simulink model "HvacPartRecircCtrl"  (R2022b)
settings: Solver=FixedStepDiscrete  StartTime=0.0  StopTime=10.0  FixedStep=0.2  RelTol=1e-3
settings Model: Creator=blue.ge  LastModifiedBy=blue.ge
settings DataIOCC: SaveFormat=StructureWithTime
settings OptimizationCC: DefaultParameterBehavior=Inlined  EnhancedBackFolding=on  PassReuseOutputArgsAs=Individual arguments  ZeroExternalMemoryAtStartup=off  ZeroInternalMemoryAtStartup=off  OptimizeBlockOrder=speed  DifferentSizesBufferReuse=on  OptimizationCustomize=off  EfficientTunableParamExpr=on
settings DebuggingCC: ParameterTunabilityLossMsg=error
settings ModelReferenceCC: ModelReferenceNumInstancesAllowed=Single
settings SFSimCC: MATLABDynamicMemAlloc=off
settings RTWCC: Description=AUTOSAR  SystemTargetFile=autosar.tlc  GenCodeOnly=on  TemplateMakefile=ert_default_tmf
settings CodeAppCC: ForceParamTrailComments=on  IgnoreCustomStorageClasses=off  OperatorAnnotations=on  SimulinkDataObjDesc=on  SFDataObjDesc=on  InsertBlockDesc=on
settings STFCustomTargetCC: TargetLangStandard=C99 (ISO)  MultiwordLength=256  ParMdlRefBuildCompliant=on  CompOptLevelCompliant=on  IncludeMdlTerminateFcn=off  CombineOutputUpdateFcns=on  SuppressErrorStatus=on  ERTCustomFileBanners=on  SupportNonFinite=off  SupportComplex=off  SupportContinuousTime=off  SupportNonInlinedSFcns=off  RemoveResetFunc=on  MATLABClassNameForMDSCustomization=Simulink.SoftwareTarget.ERTCustomization  AutosarCompliant=on  UseToolchainInfoCompliant=on  LookupTableObjectStructAxisOrder=2,1,3,4,...  LUTObjectStructOrderEvenSpacing=Size,Table,Breakpoints  SystemTargetFile=autosar.tlc
settings ERTTargetCC: TargetLangStandard=C99 (ISO)  ConcurrentExecutionCompliant=off  IncludeMdlTerminateFcn=off  SuppressErrorStatus=on  SupportNonFinite=off  SupportComplex=off  ModelStepFunctionPrototypeControlCompliant=off  CPPClassGenCompliant=off  AutosarCompliant=on  LookupTableObjectStructAxisOrder=2,1,3,4,...  LUTObjectStructOrderEvenSpacing=Size,Table,Breakpoints  InlinedParameterPlacement=NonHierarchical  ERTCustomFileTemplate=example_file_process.tlc

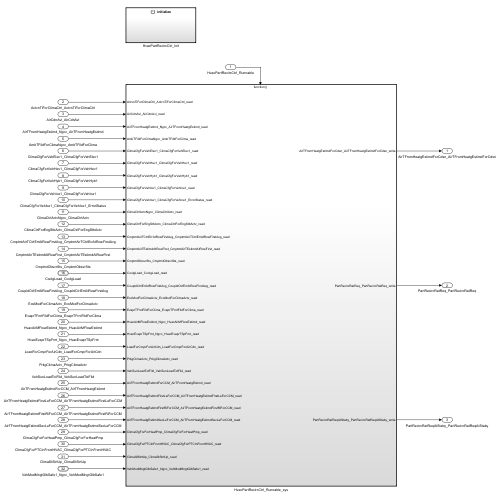
[thumbnail: Simulink's stored preview of the top-level diagram]
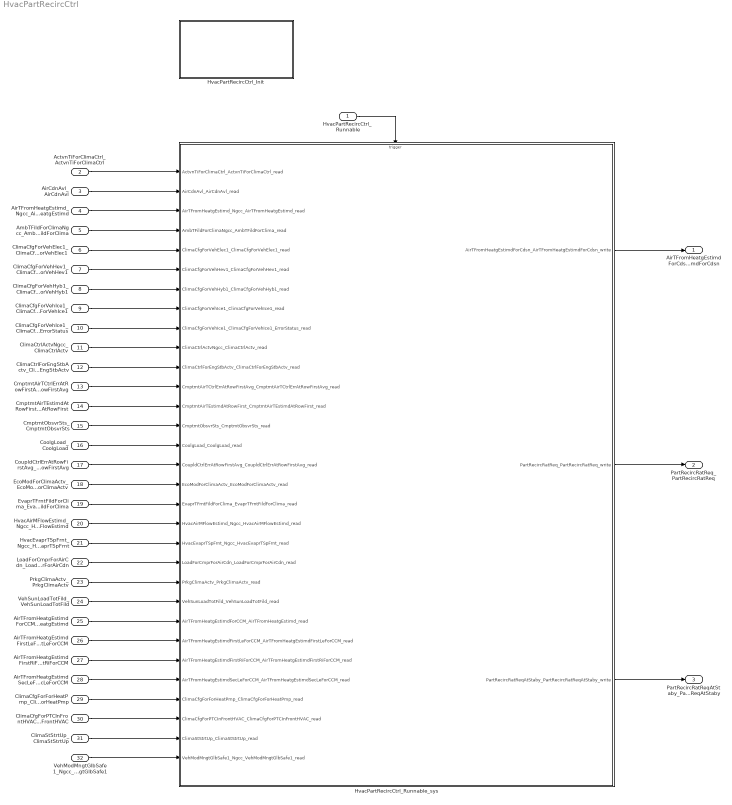
== system HvacPartRecircCtrl ==
  HvacPartRecircCtrl_Runnable : Inport  SampleTime=0.2  OutputFunctionCall=on
  ActvnTiForClimaCtrl_ActvnTiForClimaCtrl : Inport  Port=2  OutDataTypeStr=Ti33  PortDimensions=1
  AirCdnAvl_AirCdnAvl : Inport  Port=3  OutDataTypeStr=Boolean  PortDimensions=1
  AirTFromHeatgEstimd_Ngcc_AirTFromHeatgEstimd : Inport  Port=4  OutDataTypeStr=T30  PortDimensions=1
  AmbTFildForClimaNgcc_AmbTFildForClima : Inport  Port=5  OutDataTypeStr=T30  PortDimensions=1
  ClimaCfgForVehElec1_ClimaCfgForVehElec1 : Inport  Port=6  OutDataTypeStr=Boolean  PortDimensions=1
  ClimaCfgForVehHev1_ClimaCfgForVehHev1 : Inport  Port=7  OutDataTypeStr=Boolean  PortDimensions=1
  ClimaCfgForVehHyb1_ClimaCfgForVehHyb1 : Inport  Port=8  OutDataTypeStr=Boolean  PortDimensions=1
  ClimaCfgForVehIce1_ClimaCfgForVehIce1 : Inport  Port=9  OutDataTypeStr=Boolean  PortDimensions=1
  ClimaCfgForVehIce1_ClimaCfgForVehIce1_ErrorStatus : Inport  Port=10  OutDataTypeStr=uint8  PortDimensions=1
  ClimaCtrlActvNgcc_ClimaCtrlActv : Inport  Port=11  OutDataTypeStr=Boolean  PortDimensions=1
  ClimaCtrlForEngStbActv_ClimaCtrlForEngStbActv : Inport  Port=12  OutDataTypeStr=Boolean  PortDimensions=1
  CmptmtAirTCtrlErrAtRowFirstAvg_CmptmtAirTCtrlErrAtRowFirstAvg : Inport  Port=13  OutDataTypeStr=T30  PortDimensions=1
  CmptmtAirTEstimdAtRowFirst_CmptmtAirTEstimdAtRowFirst : Inport  Port=14  OutDataTypeStr=T30  PortDimensions=1
  CmptmtObsvrSts_CmptmtObsvrSts : Inport  Port=15  OutDataTypeStr=Enum: ObsvrStsForCmptmt  PortDimensions=1
  CoolgLoad_CoolgLoad : Inport  Port=16  OutDataTypeStr=Bus: CoolgLoad1  PortDimensions=1  BusOutputAsStruct=on
  CoupldCtrlErrAtRowFirstAvg_CoupldCtrlErrAtRowFirstAvg : Inport  Port=17  OutDataTypeStr=T30  PortDimensions=1
  EcoModForClimaActv_EcoModForClimaActv : Inport  Port=18  OutDataTypeStr=Enum: OnOff1  PortDimensions=1
  EvaprTFrntFildForClima_EvaprTFrntFildForClima : Inport  Port=19  OutDataTypeStr=T30  PortDimensions=1
  HvacAirMFlowEstimd_Ngcc_HvacAirMFlowEstimd : Inport  Port=20  OutMin=0  OutMax=1000  OutDataTypeStr=MFlow1  PortDimensions=1
  HvacEvaprTSpFrnt_Ngcc_HvacEvaprTSpFrnt : Inport  Port=21  OutDataTypeStr=HvacEvaprTSp  PortDimensions=1
  LoadForCmprForAirCdn_LoadForCmprForAirCdn : Inport  Port=22  OutDataTypeStr=Perc4  PortDimensions=1
  PrkgClimaActv_PrkgClimaActv : Inport  Port=23  OutDataTypeStr=Enum: PrkgClimaActv  PortDimensions=1
  VehSunLoadTotFild_VehSunLoadTotFild : Inport  Port=24  OutDataTypeStr=SunLoad30  PortDimensions=1
  AirTFromHeatgEstimdForCCM_AirTFromHeatgEstimd : Inport  Port=25  OutDataTypeStr=T30  PortDimensions=1
  AirTFromHeatgEstimdFirstLeForCCM_AirTFromHeatgEstimdFirstLeForCCM : Inport  Port=26  OutDataTypeStr=T30  PortDimensions=1
  AirTFromHeatgEstimdFirstRiForCCM_AirTFromHeatgEstimdFirstRiForCCM : Inport  Port=27  OutDataTypeStr=T30  PortDimensions=1
  AirTFromHeatgEstimdSecLeForCCM_AirTFromHeatgEstimdSecLeForCCM : Inport  Port=28  OutDataTypeStr=T30  PortDimensions=1
  ClimaCfgForForHeatPmp_ClimaCfgForForHeatPmp : Inport  Port=29  OutDataTypeStr=Enum: CarCfgForHeatPmpTyp  PortDimensions=1
  ClimaCfgForPTCInFrontHVAC_ClimaCfgForPTCInFrontHVAC : Inport  Port=30  OutDataTypeStr=Enum: FrontHeaterExchanger  PortDimensions=1
  ClimaStStrtUp_ClimaStStrtUp : Inport  Port=31  OutDataTypeStr=Enum: ClimaStStrtUp  PortDimensions=1
  VehModMngtGlbSafe1_Ngcc_VehModMngtGlbSafe1 : Inport  Port=32  OutDataTypeStr=Bus: VehModMngtGlbSafe1  PortDimensions=1  BusOutputAsStruct=on
  HvacPartRecircCtrl_Init : Subsystem
  HvacPartRecircCtrl_Runnable_sys : Subsystem
  AirTFromHeatgEstimdForCdsn_AirTFromHeatgEstimdForCdsn : Outport  OutDataTypeStr=T30  PortDimensions=1
  PartRecircRatReq_PartRecircRatReq : Outport  Port=2  OutDataTypeStr=Perc30  PortDimensions=1
  PartRecircRatReqAtStaby_PartRecircRatReqAtStaby : Outport  Port=3  OutDataTypeStr=Perc30  PortDimensions=1
  wires:
  ActvnTiForClimaCtrl_ActvnTiForClimaCtrl/1 -> HvacPartRecircCtrl_Runnable_sys/1(ActvnTiForClimaCtrl_ActvnTiForClimaCtrl_read)
  AirCdnAvl_AirCdnAvl/1 -> HvacPartRecircCtrl_Runnable_sys/2(AirCdnAvl_AirCdnAvl_read)
  AirTFromHeatgEstimd_Ngcc_AirTFromHeatgEstimd/1 -> HvacPartRecircCtrl_Runnable_sys/3(AirTFromHeatgEstimd_Ngcc_AirTFromHeatgEstimd_read)
  AmbTFildForClimaNgcc_AmbTFildForClima/1 -> HvacPartRecircCtrl_Runnable_sys/4(AmbTFildForClimaNgcc_AmbTFildForClima_read)
  ClimaCfgForVehElec1_ClimaCfgForVehElec1/1 -> HvacPartRecircCtrl_Runnable_sys/5(ClimaCfgForVehElec1_ClimaCfgForVehElec1_read)
  ClimaCfgForVehHev1_ClimaCfgForVehHev1/1 -> HvacPartRecircCtrl_Runnable_sys/6(ClimaCfgForVehHev1_ClimaCfgForVehHev1_read)
  ClimaCfgForVehHyb1_ClimaCfgForVehHyb1/1 -> HvacPartRecircCtrl_Runnable_sys/7(ClimaCfgForVehHyb1_ClimaCfgForVehHyb1_read)
  ClimaCfgForVehIce1_ClimaCfgForVehIce1/1 -> HvacPartRecircCtrl_Runnable_sys/8(ClimaCfgForVehIce1_ClimaCfgForVehIce1_read)
  ClimaCfgForVehIce1_ClimaCfgForVehIce1_ErrorStatus/1 -> HvacPartRecircCtrl_Runnable_sys/9(ClimaCfgForVehIce1_ClimaCfgForVehIce1_ErrorStatus_read)
  ClimaCtrlActvNgcc_ClimaCtrlActv/1 -> HvacPartRecircCtrl_Runnable_sys/10(ClimaCtrlActvNgcc_ClimaCtrlActv_read)
  ClimaCtrlForEngStbActv_ClimaCtrlForEngStbActv/1 -> HvacPartRecircCtrl_Runnable_sys/11(ClimaCtrlForEngStbActv_ClimaCtrlForEngStbActv_read)
  CmptmtAirTCtrlErrAtRowFirstAvg_CmptmtAirTCtrlErrAtRowFirstAvg/1 -> HvacPartRecircCtrl_Runnable_sys/12(CmptmtAirTCtrlErrAtRowFirstAvg_CmptmtAirTCtrlErrAtRowFirstAvg_read)
  CmptmtAirTEstimdAtRowFirst_CmptmtAirTEstimdAtRowFirst/1 -> HvacPartRecircCtrl_Runnable_sys/13(CmptmtAirTEstimdAtRowFirst_CmptmtAirTEstimdAtRowFirst_read)
  CmptmtObsvrSts_CmptmtObsvrSts/1 -> HvacPartRecircCtrl_Runnable_sys/14(CmptmtObsvrSts_CmptmtObsvrSts_read)
  CoolgLoad_CoolgLoad/1 -> HvacPartRecircCtrl_Runnable_sys/15(CoolgLoad_CoolgLoad_read)
  CoupldCtrlErrAtRowFirstAvg_CoupldCtrlErrAtRowFirstAvg/1 -> HvacPartRecircCtrl_Runnable_sys/16(CoupldCtrlErrAtRowFirstAvg_CoupldCtrlErrAtRowFirstAvg_read)
  EcoModForClimaActv_EcoModForClimaActv/1 -> HvacPartRecircCtrl_Runnable_sys/17(EcoModForClimaActv_EcoModForClimaActv_read)
  EvaprTFrntFildForClima_EvaprTFrntFildForClima/1 -> HvacPartRecircCtrl_Runnable_sys/18(EvaprTFrntFildForClima_EvaprTFrntFildForClima_read)
  HvacAirMFlowEstimd_Ngcc_HvacAirMFlowEstimd/1 -> HvacPartRecircCtrl_Runnable_sys/19(HvacAirMFlowEstimd_Ngcc_HvacAirMFlowEstimd_read)
  HvacEvaprTSpFrnt_Ngcc_HvacEvaprTSpFrnt/1 -> HvacPartRecircCtrl_Runnable_sys/20(HvacEvaprTSpFrnt_Ngcc_HvacEvaprTSpFrnt_read)
  LoadForCmprForAirCdn_LoadForCmprForAirCdn/1 -> HvacPartRecircCtrl_Runnable_sys/21(LoadForCmprForAirCdn_LoadForCmprForAirCdn_read)
  PrkgClimaActv_PrkgClimaActv/1 -> HvacPartRecircCtrl_Runnable_sys/22(PrkgClimaActv_PrkgClimaActv_read)
  VehSunLoadTotFild_VehSunLoadTotFild/1 -> HvacPartRecircCtrl_Runnable_sys/23(VehSunLoadTotFild_VehSunLoadTotFild_read)
  AirTFromHeatgEstimdForCCM_AirTFromHeatgEstimd/1 -> HvacPartRecircCtrl_Runnable_sys/24(AirTFromHeatgEstimdForCCM_AirTFromHeatgEstimd_read)
  AirTFromHeatgEstimdFirstLeForCCM_AirTFromHeatgEstimdFirstLeForCCM/1 -> HvacPartRecircCtrl_Runnable_sys/25(AirTFromHeatgEstimdFirstLeForCCM_AirTFromHeatgEstimdFirstLeForCCM_read)
  AirTFromHeatgEstimdFirstRiForCCM_AirTFromHeatgEstimdFirstRiForCCM/1 -> HvacPartRecircCtrl_Runnable_sys/26(AirTFromHeatgEstimdFirstRiForCCM_AirTFromHeatgEstimdFirstRiForCCM_read)
  AirTFromHeatgEstimdSecLeForCCM_AirTFromHeatgEstimdSecLeForCCM/1 -> HvacPartRecircCtrl_Runnable_sys/27(AirTFromHeatgEstimdSecLeForCCM_AirTFromHeatgEstimdSecLeForCCM_read)
  ClimaCfgForForHeatPmp_ClimaCfgForForHeatPmp/1 -> HvacPartRecircCtrl_Runnable_sys/28(ClimaCfgForForHeatPmp_ClimaCfgForForHeatPmp_read)
  ClimaCfgForPTCInFrontHVAC_ClimaCfgForPTCInFrontHVAC/1 -> HvacPartRecircCtrl_Runnable_sys/29(ClimaCfgForPTCInFrontHVAC_ClimaCfgForPTCInFrontHVAC_read)
  ClimaStStrtUp_ClimaStStrtUp/1 -> HvacPartRecircCtrl_Runnable_sys/30(ClimaStStrtUp_ClimaStStrtUp_read)
  VehModMngtGlbSafe1_Ngcc_VehModMngtGlbSafe1/1 -> HvacPartRecircCtrl_Runnable_sys/31(VehModMngtGlbSafe1_Ngcc_VehModMngtGlbSafe1_read)
  HvacPartRecircCtrl_Runnable_sys/1(AirTFromHeatgEstimdForCdsn_AirTFromHeatgEstimdForCdsn_write) -> AirTFromHeatgEstimdForCdsn_AirTFromHeatgEstimdForCdsn/1
  HvacPartRecircCtrl_Runnable_sys/2(PartRecircRatReq_PartRecircRatReq_write) -> PartRecircRatReq_PartRecircRatReq/1
  HvacPartRecircCtrl_Runnable_sys/3(PartRecircRatReqAtStaby_PartRecircRatReqAtStaby_write) -> PartRecircRatReqAtStaby_PartRecircRatReqAtStaby/1
  HvacPartRecircCtrl_Runnable/1 -> HvacPartRecircCtrl_Runnable_sys/Trigger

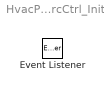
== system HvacPartRecircCtrl/HvacPartRecircCtrl_Init ==
  Event Listener : EventListener  EventName=reset

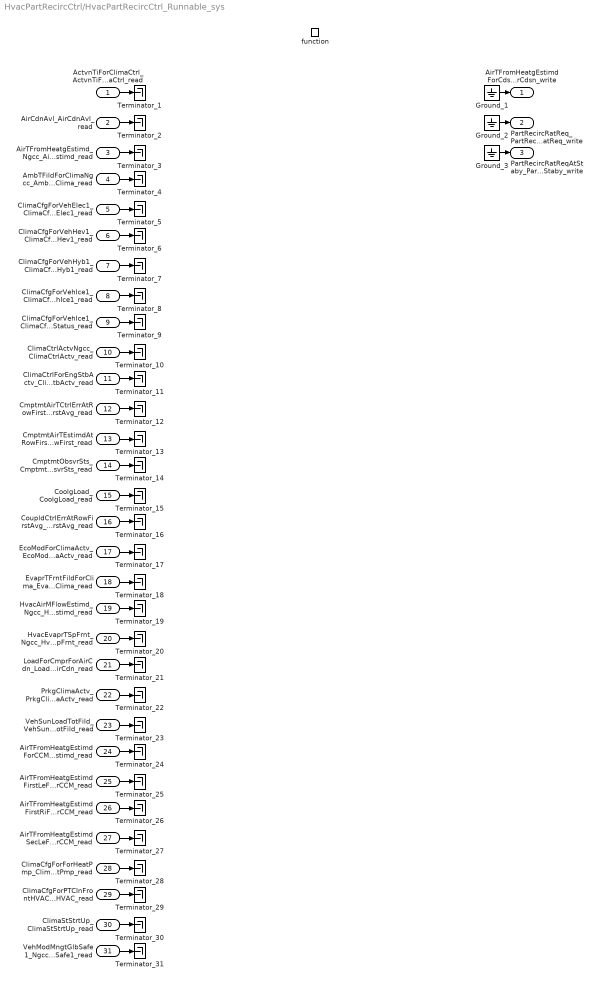
== system HvacPartRecircCtrl/HvacPartRecircCtrl_Runnable_sys ==
  ActvnTiForClimaCtrl_ActvnTiForClimaCtrl_read : Inport
  AirCdnAvl_AirCdnAvl_read : Inport  Port=2
  AirTFromHeatgEstimd_Ngcc_AirTFromHeatgEstimd_read : Inport  Port=3
  AmbTFildForClimaNgcc_AmbTFildForClima_read : Inport  Port=4
  ClimaCfgForVehElec1_ClimaCfgForVehElec1_read : Inport  Port=5
  ClimaCfgForVehHev1_ClimaCfgForVehHev1_read : Inport  Port=6
  ClimaCfgForVehHyb1_ClimaCfgForVehHyb1_read : Inport  Port=7
  ClimaCfgForVehIce1_ClimaCfgForVehIce1_read : Inport  Port=8
  ClimaCfgForVehIce1_ClimaCfgForVehIce1_ErrorStatus_read : Inport  Port=9
  ClimaCtrlActvNgcc_ClimaCtrlActv_read : Inport  Port=10
  ClimaCtrlForEngStbActv_ClimaCtrlForEngStbActv_read : Inport  Port=11
  CmptmtAirTCtrlErrAtRowFirstAvg_CmptmtAirTCtrlErrAtRowFirstAvg_read : Inport  Port=12
  CmptmtAirTEstimdAtRowFirst_CmptmtAirTEstimdAtRowFirst_read : Inport  Port=13
  CmptmtObsvrSts_CmptmtObsvrSts_read : Inport  Port=14
  CoolgLoad_CoolgLoad_read : Inport  Port=15
  CoupldCtrlErrAtRowFirstAvg_CoupldCtrlErrAtRowFirstAvg_read : Inport  Port=16
  EcoModForClimaActv_EcoModForClimaActv_read : Inport  Port=17
  EvaprTFrntFildForClima_EvaprTFrntFildForClima_read : Inport  Port=18
  HvacAirMFlowEstimd_Ngcc_HvacAirMFlowEstimd_read : Inport  Port=19
  HvacEvaprTSpFrnt_Ngcc_HvacEvaprTSpFrnt_read : Inport  Port=20
  LoadForCmprForAirCdn_LoadForCmprForAirCdn_read : Inport  Port=21
  PrkgClimaActv_PrkgClimaActv_read : Inport  Port=22
  VehSunLoadTotFild_VehSunLoadTotFild_read : Inport  Port=23
  AirTFromHeatgEstimdForCCM_AirTFromHeatgEstimd_read : Inport  Port=24
  AirTFromHeatgEstimdFirstLeForCCM_AirTFromHeatgEstimdFirstLeForCCM_read : Inport  Port=25
  AirTFromHeatgEstimdFirstRiForCCM_AirTFromHeatgEstimdFirstRiForCCM_read : Inport  Port=26
  AirTFromHeatgEstimdSecLeForCCM_AirTFromHeatgEstimdSecLeForCCM_read : Inport  Port=27
  ClimaCfgForForHeatPmp_ClimaCfgForForHeatPmp_read : Inport  Port=28
  ClimaCfgForPTCInFrontHVAC_ClimaCfgForPTCInFrontHVAC_read : Inport  Port=29
  ClimaStStrtUp_ClimaStStrtUp_read : Inport  Port=30
  VehModMngtGlbSafe1_Ngcc_VehModMngtGlbSafe1_read : Inport  Port=31
  function : TriggerPort  TriggerType=function-call  VariantControl=(inherit)  SampleTimeType=periodic  SampleTime=-1
  Ground_1 : Ground
  Ground_2 : Ground
  Ground_3 : Ground
  Terminator_{1..31} (31 alike) : Terminator
  AirTFromHeatgEstimdForCdsn_AirTFromHeatgEstimdForCdsn_write : Outport
      settings: EnsureOutportIsVirtual=on
  PartRecircRatReq_PartRecircRatReq_write : Outport  Port=2
      settings: EnsureOutportIsVirtual=on
  PartRecircRatReqAtStaby_PartRecircRatReqAtStaby_write : Outport  Port=3
      settings: EnsureOutportIsVirtual=on
  wires:
  ActvnTiForClimaCtrl_ActvnTiForClimaCtrl_read/1 -> Terminator_1/1
  AirCdnAvl_AirCdnAvl_read/1 -> Terminator_2/1
  AirTFromHeatgEstimd_Ngcc_AirTFromHeatgEstimd_read/1 -> Terminator_3/1
  AmbTFildForClimaNgcc_AmbTFildForClima_read/1 -> Terminator_4/1
  ClimaCfgForVehElec1_ClimaCfgForVehElec1_read/1 -> Terminator_5/1
  ClimaCfgForVehHev1_ClimaCfgForVehHev1_read/1 -> Terminator_6/1
  ClimaCfgForVehHyb1_ClimaCfgForVehHyb1_read/1 -> Terminator_7/1
  ClimaCfgForVehIce1_ClimaCfgForVehIce1_read/1 -> Terminator_8/1
  ClimaCfgForVehIce1_ClimaCfgForVehIce1_ErrorStatus_read/1 -> Terminator_9/1
  ClimaCtrlActvNgcc_ClimaCtrlActv_read/1 -> Terminator_10/1
  ClimaCtrlForEngStbActv_ClimaCtrlForEngStbActv_read/1 -> Terminator_11/1
  CmptmtAirTCtrlErrAtRowFirstAvg_CmptmtAirTCtrlErrAtRowFirstAvg_read/1 -> Terminator_12/1
  CmptmtAirTEstimdAtRowFirst_CmptmtAirTEstimdAtRowFirst_read/1 -> Terminator_13/1
  CmptmtObsvrSts_CmptmtObsvrSts_read/1 -> Terminator_14/1
  CoolgLoad_CoolgLoad_read/1 -> Terminator_15/1
  CoupldCtrlErrAtRowFirstAvg_CoupldCtrlErrAtRowFirstAvg_read/1 -> Terminator_16/1
  EcoModForClimaActv_EcoModForClimaActv_read/1 -> Terminator_17/1
  EvaprTFrntFildForClima_EvaprTFrntFildForClima_read/1 -> Terminator_18/1
  HvacAirMFlowEstimd_Ngcc_HvacAirMFlowEstimd_read/1 -> Terminator_19/1
  HvacEvaprTSpFrnt_Ngcc_HvacEvaprTSpFrnt_read/1 -> Terminator_20/1
  LoadForCmprForAirCdn_LoadForCmprForAirCdn_read/1 -> Terminator_21/1
  PrkgClimaActv_PrkgClimaActv_read/1 -> Terminator_22/1
  VehSunLoadTotFild_VehSunLoadTotFild_read/1 -> Terminator_23/1
  AirTFromHeatgEstimdForCCM_AirTFromHeatgEstimd_read/1 -> Terminator_24/1
  AirTFromHeatgEstimdFirstLeForCCM_AirTFromHeatgEstimdFirstLeForCCM_read/1 -> Terminator_25/1
  AirTFromHeatgEstimdFirstRiForCCM_AirTFromHeatgEstimdFirstRiForCCM_read/1 -> Terminator_26/1
  AirTFromHeatgEstimdSecLeForCCM_AirTFromHeatgEstimdSecLeForCCM_read/1 -> Terminator_27/1
  ClimaCfgForForHeatPmp_ClimaCfgForForHeatPmp_read/1 -> Terminator_28/1
  ClimaCfgForPTCInFrontHVAC_ClimaCfgForPTCInFrontHVAC_read/1 -> Terminator_29/1
  ClimaStStrtUp_ClimaStStrtUp_read/1 -> Terminator_30/1
  VehModMngtGlbSafe1_Ngcc_VehModMngtGlbSafe1_read/1 -> Terminator_31/1
  Ground_1/1 -> AirTFromHeatgEstimdForCdsn_AirTFromHeatgEstimdForCdsn_write/1
  Ground_2/1 -> PartRecircRatReq_PartRecircRatReq_write/1
  Ground_3/1 -> PartRecircRatReqAtStaby_PartRecircRatReqAtStaby_write/1
